simulink model "wlan_phy_rx_pmd"  (Simulink 7.8)
settings: Solver=VariableStepDiscrete  SolverMode=SingleTasking  StartTime=0.0  StopTime=rx_sim_time  RelTol=1e-3
PostLoadFcn:
  | wlan_phy_rx_init
InitFcn:
  | wlan_phy_rx_init
StartFcn:
  | wlan_phy_rx_init
values from neighbouring files (symbols this model uses):
  barker_seq20 = 0.95 * circshift(barker_seq20, [0 4]) ./ max(abs(barker_seq20))    [wlan_phy_rx_init.m]
  bit_scrambler_lfsr_bytes = sum(bit_scrambler_lfsr_bytes .* repmat(2.^[0:7], 127, 1),2)    [wlan_phy_rx_init.m]
  deinterleave_ROM = [deinterleave_ROM interleave_11n_64QAM zeros(1, 512-length(interleave_11n_64QAM))]    [wlan_phy_rx_init.m]
  longCorr_coef_i = [3*real(longCorr_coef)]    [wlan_phy_rx_init.m]
  longCorr_coef_q = [3*imag(longCorr_coef)]    [wlan_phy_rx_init.m]
  MAX_CP_LEN = 32    [wlan_phy_rx_init.m]
  MAX_NCBPS = NCBPS_11n_64QAM    (= 312)    [wlan_phy_rx_init.m]
  MAX_NUM_BYTES = 2^16-1    (= 65535)    [wlan_phy_rx_init.m]
  MAX_NUM_SC = 64    [wlan_phy_rx_init.m]
  MAX_NUM_SYMS = 600    [wlan_phy_rx_init.m]
  NCBPS_11a_BPSK = 48    [wlan_phy_rx_init.m]
  NDBPS_11a_BPSK12 = 24    [wlan_phy_rx_init.m]
  PHY_SIGNAL_MIN_LEN = 14    [wlan_phy_rx_init.m]
  preFFT_sampBuff_numSamps = 4*MAX_NUM_SC    (= 256)    [wlan_phy_rx_init.m]
  rx_sim_sig_samp_time = 8    [wlan_phy_rx_init.m]
  scr_ind_rev = zeros(1,128)    [wlan_phy_rx_init.m]
undefined symbols (not defined in the file or in its folder): gcbh, mcs_rom_11ag, mcs_rom_11n, xlBlockLoadCallback, xlGetNormalizedPeriod, xlipgetpartsetting
libraries: xbsIndex_r4 x3862, simulink x1, xbsEDKLib_r4 x1
(part 3 of 23)

== system wlan_phy_rx_pmd/FCS & Pkt Buf ==
  DSSS data : Inport
  OFDM data : Inport  Port=2
  Bus Selector : BusSelector  OutputSignals=byte_valid,byte_index,byte,last_byte
  Bus Selector1 : BusSelector  OutputSignals=byte_valid,byte_index,byte,last_byte
  Bytes In : Scope  NumInputPorts=8
      settings: TimeRange=10000  YMin=0~0~0~-1~-1~-1~-1~-1  YMax=1~90~225~1~1~1~1~1  SaveName=ScopeData36
  FCS Calc : Subsystem
  Gateway Out{1..4,8..9,11..12} (8 alike) : xbsIndex_r4/Gateway Out  inherit_from_input=off  hdl_port=off  timing_constraint=None  locs_specified=off  LOCs={}  UseAsDAC=off  DACChannel='1'  block_type=gatewayout
  Pkt Buf Interface : Subsystem
  wires:
  OFDM data/1 -> Bus Selector/1, Pkt Buf Interface/2(OFDM data), FCS Calc/2(OFDM data)
  DSSS data/1 -> Bus Selector1/1, Pkt Buf Interface/1(DSSS data), FCS Calc/1(DSSS data)
  Bus Selector1/1 -> Gateway Out9/1  "<byte_valid>"
  Bus Selector1/2 -> Gateway Out8/1  "<byte_index>"
  Bus Selector1/3 -> Gateway Out11/1  "<byte>"
  Bus Selector1/4 -> Gateway Out12/1  "<last_byte>"
  Bus Selector/1 -> Gateway Out1/1  "<byte_valid>"
  Bus Selector/2 -> Gateway Out2/1  "<byte_index>"
  Bus Selector/3 -> Gateway Out3/1  "<byte>"
  Bus Selector/4 -> Gateway Out4/1  "<last_byte>"
  Gateway Out9/1 -> Bytes In/1  "DSSS Valid"
  Gateway Out8/1 -> Bytes In/2  "DSSS Byte Index"
  Gateway Out11/1 -> Bytes In/3  "DSSS Byte"
  Gateway Out12/1 -> Bytes In/4  "DSSS Last Byte"
  Gateway Out1/1 -> Bytes In/5  "OFDM Valid"
  Gateway Out2/1 -> Bytes In/6  "OFDM Byte Index"
  Gateway Out3/1 -> Bytes In/7  "OFDM Byte"
  Gateway Out4/1 -> Bytes In/8  "OFDM Last Byte"

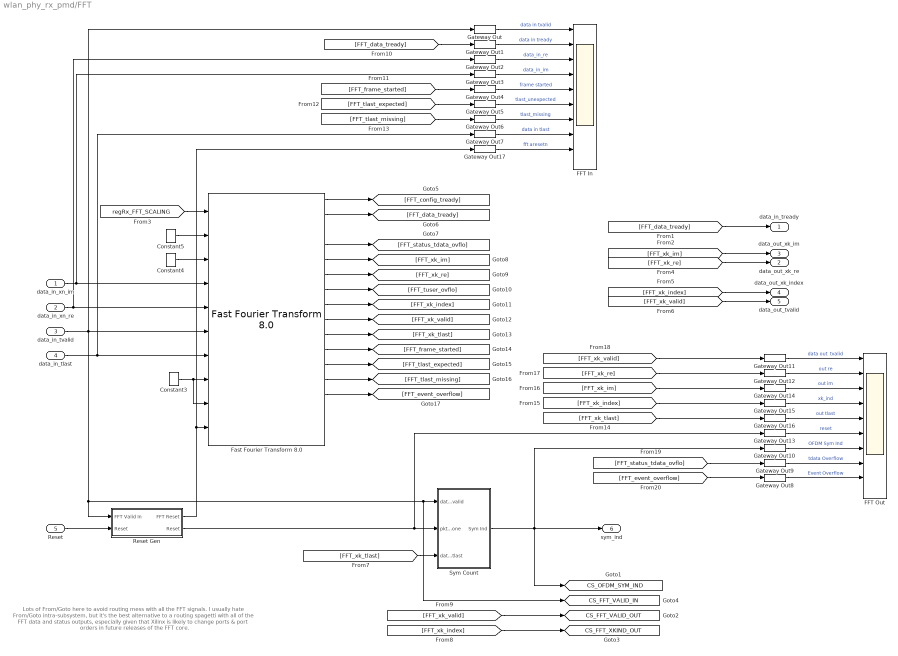
== system wlan_phy_rx_pmd/FFT ==
  note: Lots of From/Goto here to avoid routing
      | mess with all the FFT signals. I usually hate
      | From/Goto intra-subsystem, but it's the best
      | alternative to a routing spagetti with all of the
      | FFT data and status outputs, especially given
      | that Xilinx is likely to change ports & port orders
      | in future releases of the FFT core.
  data_in_xn_im : Inport
  data_in_xn_re : Inport  Port=2
  data_in_tvalid : Inport  Port=3
  data_in_tlast : Inport  Port=4
  Reset : Inport  Port=5
  Constant3 : xbsIndex_r4/Constant  const=1  gui_display_data_type=Boolean  arith_type=Boolean  n_bits=1  bin_pt=0  preci_type=Single  exp_width=8  frac_width=24  explicit_period=on  period=1  equ=P=C  opselect=C  inp2=PCIN>>17  opr=+  inp1=P  carry=CIN  dbl_ovrd=off  block_type=constant
  Constant4 : xbsIndex_r4/Constant  const=1  gui_display_data_type=Boolean  arith_type=Boolean  n_bits=1  bin_pt=0  preci_type=Single  exp_width=8  frac_width=24  explicit_period=on  period=1  equ=P=C  opselect=C  inp2=PCIN>>17  opr=+  inp1=P  carry=CIN  dbl_ovrd=off  block_type=constant
  Constant5 : xbsIndex_r4/Constant  const=1  gui_display_data_type=Fixed-point  arith_type=Unsigned  n_bits=1  bin_pt=0  preci_type=Single  exp_width=8  frac_width=24  explicit_period=on  period=1  equ=P=C  opselect=C  inp2=PCIN>>17  opr=+  inp1=P  carry=CIN  dbl_ovrd=off  block_type=constant
  FFT In : Scope  NumInputPorts=10
      settings: TimeRange=5000  YMin=0~0~-0.2~-1~0~-1~0~-1~-5~-5  YMax=1~1~0.8~1~1~1~1~1~5~5
  FFT Out : Scope  NumInputPorts=10
      settings: TimeRange=5000  YMin=0~-0.4~-0.4~0~0~-1~0~-1~-5~-5  YMax=1~0.4~0.4~70~1~1~1~1~5~5  SaveName=ScopeData29
  "Fast Fourier Transform 8.0 " : xbsIndex_r4/Fast Fourier Transform 8.0   transform_length=64  target_clock_frequency=160  target_data_throughput=50  implementation_options=radix_4_burst_io  run_time_configurable_transform_length=off  phase_factor_width=16  scaling_options=scaled  rounding_modes=truncation  aclken=off  aresetn=on  cyclic_prefix_insertion=off  output_ordering=natural_order  throttle_scheme=nonrealtime  xk_index=on  ovflo=on  trim_axipin_name=on  memory_options_data=block_ram  memory_options_phase_factors=block_ram  number_of_stages_using_block_ram_for_data_and_phase_factors=0  memory_options_reorder=block_ram  memory_options_hybrid=off  complex_mult_type=use_mults_performance  butterfly_type=use_luts  channels=1  input_ordering=natural_order  input_width=16  ip_name=Fast Fourier Transform  ip_version=8.0  dsptool_ready=true  ipcore_usecache=true  ipcore_useipmodelcache=true  wrapper_available=true  port_translation_map={ 'aclken' => 'en', 'aresetn' => 'rst'}  block_type=xfft_v8_0
  Gateway Out{,1..17} (18 alike) : xbsIndex_r4/Gateway Out  inherit_from_input=off  hdl_port=off  timing_constraint=None  locs_specified=off  LOCs={}  UseAsDAC=off  DACChannel='1'  block_type=gatewayout
  Reset Gen : Subsystem
  Sym Count : Subsystem
  data_in_tready : Outport
  data_out_xk_re : Outport  Port=2
  data_out_xk_im : Outport  Port=3
  data_out_xk_index : Outport  Port=4
  data_out_tvalid : Outport  Port=5
  sym_ind : Outport  Port=6
  tag scope: CS_FFT_VALID_IN=global, CS_FFT_VALID_OUT=global, CS_FFT_XKIND_OUT=global, CS_OFDM_SYM_IND=global
  wires:
  Gateway Out13/1 -> FFT Out/6  "reset"
  Gateway Out16/1 -> FFT Out/5  "out tlast"
  Gateway Out15/1 -> FFT Out/4  "xk_ind"
  Gateway Out14/1 -> FFT Out/3  "out im"
  Gateway Out12/1 -> FFT Out/2  "out re"
  Gateway Out11/1 -> FFT Out/1  "data out  tvalid"
  [FFT_xk_index] -> data_out_xk_index/1
  [FFT_xk_re] -> data_out_xk_re/1
  [FFT_xk_tlast] -> Sym Count/3(data_out_tlast)
  [FFT_xk_valid] -> data_out_tvalid/1
  [FFT_xk_im] -> data_out_xk_im/1
  [FFT_data_tready] -> data_in_tready/1
  Gateway Out7/1 -> FFT In/8  "data in tlast"
  Gateway Out6/1 -> FFT In/7  "tlast_missing"
  Gateway Out5/1 -> FFT In/6  "tlast_unexpected"
  Gateway Out4/1 -> FFT In/5  "frame started"
  Gateway Out3/1 -> FFT In/4  "data_in_im"
  Gateway Out2/1 -> FFT In/3  "data_in_re"
  Gateway Out1/1 -> FFT In/2  "data in tready"
  Gateway Out/1 -> FFT In/1  "data in tvalid"
  "Fast Fourier Transform 8.0 "/1 -> [FFT_config_tready]
  "Fast Fourier Transform 8.0 "/2 -> [FFT_data_tready]
  "Fast Fourier Transform 8.0 "/4 -> [FFT_status_tdata_ovflo]
  "Fast Fourier Transform 8.0 "/5 -> [FFT_xk_im]
  "Fast Fourier Transform 8.0 "/6 -> [FFT_xk_re]
  "Fast Fourier Transform 8.0 "/7 -> [FFT_tuser_ovflo]
  "Fast Fourier Transform 8.0 "/8 -> [FFT_xk_index]
  "Fast Fourier Transform 8.0 "/9 -> [FFT_xk_valid]
  "Fast Fourier Transform 8.0 "/10 -> [FFT_xk_tlast]
  "Fast Fourier Transform 8.0 "/11 -> [FFT_frame_started]
  "Fast Fourier Transform 8.0 "/12 -> [FFT_tlast_expected]
  "Fast Fourier Transform 8.0 "/13 -> [FFT_tlast_missing]
  "Fast Fourier Transform 8.0 "/14 -> [FFT_event_overflow]
  Constant3/1 -> "Fast Fourier Transform 8.0 "/8, "Fast Fourier Transform 8.0 "/9
  Constant4/1 -> "Fast Fourier Transform 8.0 "/3
  Constant5/1 -> "Fast Fourier Transform 8.0 "/2
  [regRx_FFT_SCALING] -> "Fast Fourier Transform 8.0 "/1
  [FFT_xk_index] -> [CS_FFT_XKIND_OUT]
  [FFT_xk_valid] -> [CS_FFT_VALID_OUT]
  Reset Gen/2(" Reset") -> Sym Count/2(pkt_done), Gateway Out13/1
  Reset/1 -> Reset Gen/2(Reset)
  data_in_tvalid/1 -> "Fast Fourier Transform 8.0 "/6, Reset Gen/1(FFT Valid In), Sym Count/1(data_in_tvalid), [CS_FFT_VALID_IN], Gateway Out/1
  Sym Count/1(Sym Ind) -> sym_ind/1, Gateway Out10/1, [CS_OFDM_SYM_IND]
  data_in_tlast/1 -> "Fast Fourier Transform 8.0 "/7, Gateway Out7/1
  data_in_xn_im/1 -> "Fast Fourier Transform 8.0 "/4, Gateway Out3/1
  data_in_xn_re/1 -> "Fast Fourier Transform 8.0 "/5, Gateway Out2/1
  Gateway Out10/1 -> FFT Out/7  "OFDM Sym Ind"
  Gateway Out9/1 -> FFT Out/8  "tdata Overflow"
  [FFT_data_tready] -> Gateway Out1/1
  [FFT_frame_started] -> Gateway Out4/1
  [FFT_tlast_expected] -> Gateway Out5/1
  [FFT_tlast_missing] -> Gateway Out6/1
  [FFT_xk_tlast] -> Gateway Out16/1
  [FFT_xk_index] -> Gateway Out15/1
  [FFT_xk_im] -> Gateway Out14/1
  [FFT_xk_re] -> Gateway Out12/1
  [FFT_xk_valid] -> Gateway Out11/1
  [FFT_status_tdata_ovflo] -> Gateway Out9/1
  [FFT_event_overflow] -> Gateway Out8/1
  Gateway Out8/1 -> FFT Out/9  "Event Overflow"
  Reset Gen/1(FFT Reset) -> "Fast Fourier Transform 8.0 "/10, Gateway Out17/1
  Gateway Out17/1 -> FFT In/9  "fft aresetn"

== system wlan_phy_rx_pmd/Inputs ==
  ADC_RX_CLK : xbsIndex_r4/Gateway In  gui_display_data_type=Boolean  arith_type=Boolean  n_bits=1  bin_pt=0  preci_type=Single  exp_width=8  frac_width=24  quantization="Round  (unbiased: +/- Inf)"  overflow=Saturate  period=1  dbl_ovrd=off  timing_constraint=None  locs_specified=off  LOCs={}  UseAsADC=off  ADCChannel='1'  inherit_from_input=off  hdl_port=on  block_type=gatewayin
  AGC_DONE : xbsIndex_r4/Gateway In  gui_display_data_type=Boolean  arith_type=Boolean  n_bits=5  bin_pt=0  preci_type=Single  exp_width=8  frac_width=24  quantization="Round  (unbiased: +/- Inf)"  overflow=Saturate  period=1  dbl_ovrd=off  timing_constraint=None  locs_specified=off  LOCs={}  UseAsADC=off  ADCChannel='1'  inherit_from_input=off  hdl_port=on  block_type=gatewayin
  Bus Creator{,1..3} (4 alike) : BusCreator
  Constant : Constant  Value=0
  Constant1 : Constant
  Convert2 : xbsIndex_r4/Convert  gui_display_data_type=Boolean  arith_type=Boolean  n_bits=1  bin_pt=0  float_type=Single  exp_bits=8  fraction_bits=24  quantization=Truncate  overflow=Wrap  en=off  latency=0  dbl_ovrd=off  pipeline=off  block_type=convert
  Delay3 : xbsIndex_r4/Delay  rst=off  infoeditControl=Selection of Reset will increase slice count due to use of real FFs and instead of SRLs  en=off  latency=1  dbl_ovrd=off  reg_retiming=off  block_type=delay
  Logical : xbsIndex_r4/Logical  logical_function=AND  inputs=2  en=off  latency=0  precision=Full  arith_type=Unsigned  n_bits=16  bin_pt=0  align_bp=on  dbl_ovrd=off  block_type=logical
  Pulse Generator3 : DiscretePulseGenerator  Period=8
  RF A Inputs : Subsystem
  RF B Inputs : Subsystem
  RF C Inputs : Subsystem
  RF D Inputs : Subsystem
  RSSI Clock Gen : Subsystem
  RSSI_ADC_CLK : xbsIndex_r4/Gateway Out  inherit_from_input=off  hdl_port=on  timing_constraint=None  locs_specified=off  LOCs={}  UseAsDAC=off  DACChannel='1'  block_type=gatewayout
      settings: infoedit="Gateway out block.  Converts Xilinx fixed point inputs into ouputs of type Simulink integer, double, or fixed point.<P><P>Hardware notes:  In hardware these blocks become top level output ports or are discarded, depending on how they are configured."  block_version=8.2
  RX_SIGS_INVALID : xbsIndex_r4/Gateway In  gui_display_data_type=Boolean  arith_type=Boolean  n_bits=1  bin_pt=0  preci_type=Single  exp_width=8  frac_width=24  quantization="Round  (unbiased: +/- Inf)"  overflow=Saturate  period=1  dbl_ovrd=off  timing_constraint=None  locs_specified=off  LOCs={}  UseAsADC=off  ADCChannel='1'  inherit_from_input=off  hdl_port=on  block_type=gatewayin
  Register3 : xbsIndex_r4/Register  init=0  rst=off  en=off  dbl_ovrd=off  block_type=register
  Register6 : xbsIndex_r4/Register  init=0  rst=off  en=off  dbl_ovrd=off  block_type=register
  Terminator : Terminator
  negedge1 : Subsystem
  ADC I : Outport
  ADC Q : Outport  Port=2
  IQ Valid : Outport  Port=3
  RSSI : Outport  Port=4
  tag scope: AGC_DONE=global, CS_ADC_IQ_Valid=global, CS_RX_SIGS_INVALID=global
  wires:
  RSSI_ADC_CLK/1 -> Terminator/1
  RSSI Clock Gen/1(Clk) -> RSSI_ADC_CLK/1
  Constant/1 -> RX_SIGS_INVALID/1
  RX_SIGS_INVALID/1 -> Register6/1
  Logical/1 -> [CS_RX_SIGS_INVALID], RF A Inputs/1(Rst), RF B Inputs/1(Rst), RF C Inputs/1(Rst), RF D Inputs/1(Rst)
  Register6/1 -> Logical/2
  [REG_USE_RX_SIGS_INVALID_INPUT] -> Logical/1
  Bus Creator/1 -> ADC I/1
  Bus Creator1/1 -> ADC Q/1
  Bus Creator2/1 -> RSSI/1
  [RFA_ADC_I] -> Bus Creator/1  "RFA_I"
  [RFB_ADC_I] -> Bus Creator/2  "RFB_I"
  [RFA_ADC_Q] -> Bus Creator1/1  "RFA_Q"
  [RFB_ADC_Q] -> Bus Creator1/2  "RFB_Q"
  [RFA_RSSI] -> Bus Creator2/1  "RFA_RSSI"
  [RFB_RSSI] -> Bus Creator2/2  "RFB_RSSI"
  AGC_DONE/1 -> Delay3/1
  Delay3/1 -> [AGC_DONE]
  Bus Creator3/1 -> IQ Valid/1
  Convert2/1 -> [CS_ADC_IQ_Valid], RF A Inputs/2(IQ Valid), Bus Creator3/1, Bus Creator3/2, Bus Creator3/3, Bus Creator3/4, RF B Inputs/2(IQ Valid), RF C Inputs/2(IQ Valid), RF D Inputs/2(IQ Valid)
  negedge1/1(Q) -> Convert2/1
  Register3/1 -> negedge1/1(D)
  ADC_RX_CLK/1 -> Register3/1
  Pulse Generator3/1 -> ADC_RX_CLK/1
  RF A Inputs/1(ADC I) -> [RFA_ADC_I]
  RF A Inputs/2(ADC Q) -> [RFA_ADC_Q]
  RF A Inputs/3(Avg RSSI) -> [RFA_RSSI]
  RF B Inputs/1(ADC I) -> [RFB_ADC_I]
  RF B Inputs/2(ADC Q) -> [RFB_ADC_Q]
  RF B Inputs/3(Avg RSSI) -> [RFB_RSSI]
  [RFD_ADC_I] -> Bus Creator/4  "RFD_I"
  [RFC_ADC_I] -> Bus Creator/3  "RFC_I"
  [RFC_ADC_Q] -> Bus Creator1/3  "RFC_Q"
  [RFD_ADC_Q] -> Bus Creator1/4  "RFD_Q"
  [RFC_RSSI] -> Bus Creator2/3  "RFC_RSSI"
  [RFD_RSSI] -> Bus Creator2/4  "RFD_RSSI"
  RF C Inputs/1(ADC I) -> [RFC_ADC_I]
  RF C Inputs/2(ADC Q) -> [RFC_ADC_Q]
  RF C Inputs/3(Avg RSSI) -> [RFC_RSSI]
  RF D Inputs/1(ADC I) -> [RFD_ADC_I]
  RF D Inputs/2(ADC Q) -> [RFD_ADC_Q]
  RF D Inputs/3(Avg RSSI) -> [RFD_RSSI]
  Constant1/1 -> AGC_DONE/1

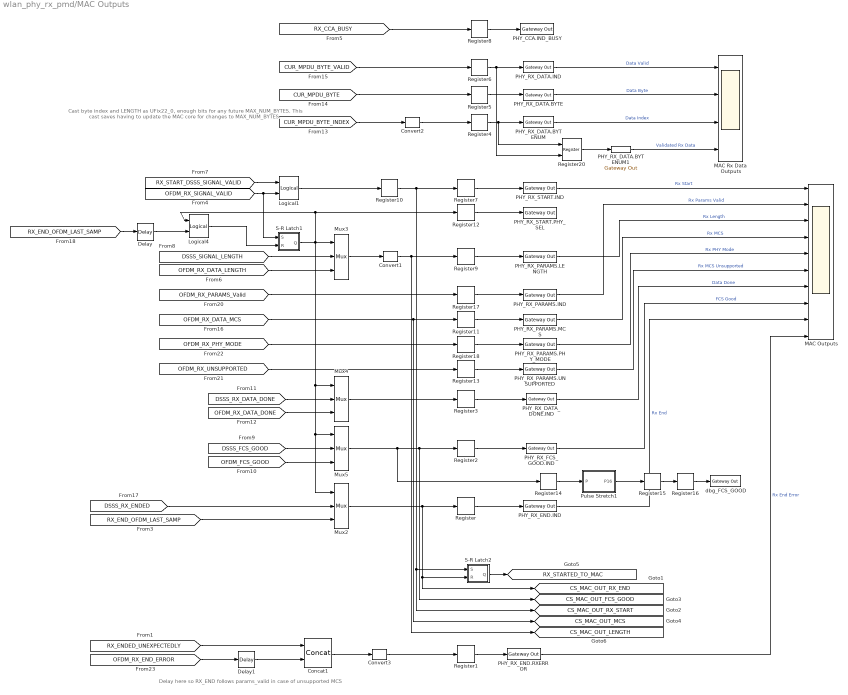
== system wlan_phy_rx_pmd/MAC Outputs ==
  note: Cast byte index and LENGTH as UFix22_0, enough bits
      | for any future MAX_NUM_BYTES. This cast saves having to
      | update the MAC core for changes to MAX_NUM_BYTES.
  note: Delay here so RX_END follows params_valid
      | in case of unsupported MCS
  Concat1 : xbsIndex_r4/Concat  num_inputs=2  dbl_ovrd=off  block_type=concat
  Convert1 : xbsIndex_r4/Convert  gui_display_data_type=Fixed-point  arith_type=Unsigned  n_bits=ceil(log2(MAX_NUM_BYTES))  bin_pt=0  float_type=Single  exp_bits=8  fraction_bits=24  quantization=Truncate  overflow=Wrap  en=off  latency=0  dbl_ovrd=off  pipeline=off  block_type=convert
  Convert2 : xbsIndex_r4/Convert  gui_display_data_type=Fixed-point  arith_type=Unsigned  n_bits=ceil(log2(MAX_NUM_BYTES))  bin_pt=0  float_type=Single  exp_bits=8  fraction_bits=24  quantization=Truncate  overflow=Wrap  en=off  latency=0  dbl_ovrd=off  pipeline=off  block_type=convert
  Convert3 : xbsIndex_r4/Convert  gui_display_data_type=Fixed-point  arith_type=Unsigned  n_bits=4  bin_pt=0  float_type=Single  exp_bits=8  fraction_bits=24  quantization=Truncate  overflow=Wrap  en=off  latency=0  dbl_ovrd=off  pipeline=off  block_type=convert
  Delay : xbsIndex_r4/Delay  rst=off  infoeditControl=Selection of Reset will increase slice count due to use of real FFs and instead of SRLs  en=off  latency=8  dbl_ovrd=off  reg_retiming=off  block_type=delay
  Delay1 : xbsIndex_r4/Delay  rst=off  infoeditControl=Selection of Reset will increase slice count due to use of real FFs and instead of SRLs  en=off  latency=2  dbl_ovrd=off  reg_retiming=off  block_type=delay
  Logical1 : xbsIndex_r4/Logical  logical_function=OR  inputs=2  en=off  latency=0  precision=Full  arith_type=Unsigned  n_bits=16  bin_pt=0  align_bp=on  dbl_ovrd=off  block_type=logical
  Logical4 : xbsIndex_r4/Logical  logical_function=AND  inputs=2  en=off  latency=0  precision=Full  arith_type=Unsigned  n_bits=16  bin_pt=0  align_bp=on  dbl_ovrd=off  block_type=logical
  MAC Outputs : Scope  NumInputPorts=10
      settings: TimeRange=9380  YMin=0~0~0~0~0~-1~0~0~0~-1  YMax=1~1~150~4~1~1~1~1~1~1  SaveName=ScopeData37
  MAC Rx Data Outputs : Scope  NumInputPorts=4
      settings: TimeRange=70000  YMin=0~0~0~0  YMax=1~1~150~8  SaveName=ScopeData32
  Mux{2..5} (4 alike) : xbsIndex_r4/Mux  inputs=2  en=off  latency=0  precision=Full  arith_type=Unsigned  n_bits=16  bin_pt=14  quantization=Truncate  overflow=Wrap  dbl_ovrd=off  block_type=mux
  PHY_CCA.IND_BUSY : xbsIndex_r4/Gateway Out  inherit_from_input=off  hdl_port=on  timing_constraint=None  locs_specified=off  LOCs={}  UseAsDAC=off  DACChannel='1'  block_type=gatewayout
  PHY_RX_DATA.BYTE : xbsIndex_r4/Gateway Out  inherit_from_input=off  hdl_port=on  timing_constraint=None  locs_specified=off  LOCs={}  UseAsDAC=off  DACChannel='1'  block_type=gatewayout
  PHY_RX_DATA.BYTENUM : xbsIndex_r4/Gateway Out  inherit_from_input=off  hdl_port=on  timing_constraint=None  locs_specified=off  LOCs={}  UseAsDAC=off  DACChannel='1'  block_type=gatewayout
  PHY_RX_DATA.BYTENUM1 : xbsIndex_r4/Gateway Out  inherit_from_input=off  hdl_port=off  timing_constraint=None  locs_specified=off  LOCs={}  UseAsDAC=off  DACChannel='1'  block_type=gatewayout
  PHY_RX_DATA.IND : xbsIndex_r4/Gateway Out  inherit_from_input=off  hdl_port=on  timing_constraint=None  locs_specified=off  LOCs={}  UseAsDAC=off  DACChannel='1'  block_type=gatewayout
  PHY_RX_DATA_DONE.IND : xbsIndex_r4/Gateway Out  inherit_from_input=off  hdl_port=on  timing_constraint=None  locs_specified=off  LOCs={}  UseAsDAC=off  DACChannel='1'  block_type=gatewayout
  PHY_RX_END.IND : xbsIndex_r4/Gateway Out  inherit_from_input=off  hdl_port=on  timing_constraint=None  locs_specified=off  LOCs={}  UseAsDAC=off  DACChannel='1'  block_type=gatewayout
  PHY_RX_END.RXERROR : xbsIndex_r4/Gateway Out  inherit_from_input=off  hdl_port=on  timing_constraint=None  locs_specified=off  LOCs={}  UseAsDAC=off  DACChannel='1'  block_type=gatewayout
  PHY_RX_FCS_GOOD.IND : xbsIndex_r4/Gateway Out  inherit_from_input=off  hdl_port=on  timing_constraint=None  locs_specified=off  LOCs={}  UseAsDAC=off  DACChannel='1'  block_type=gatewayout
  PHY_RX_PARAMS.IND : xbsIndex_r4/Gateway Out  inherit_from_input=off  hdl_port=on  timing_constraint=None  locs_specified=off  LOCs={}  UseAsDAC=off  DACChannel='1'  block_type=gatewayout
  PHY_RX_PARAMS.LENGTH : xbsIndex_r4/Gateway Out  inherit_from_input=off  hdl_port=on  timing_constraint=None  locs_specified=off  LOCs={}  UseAsDAC=off  DACChannel='1'  block_type=gatewayout
  PHY_RX_PARAMS.MCS : xbsIndex_r4/Gateway Out  inherit_from_input=off  hdl_port=on  timing_constraint=None  locs_specified=off  LOCs={}  UseAsDAC=off  DACChannel='1'  block_type=gatewayout
  PHY_RX_PARAMS.PHY_MODE : xbsIndex_r4/Gateway Out  inherit_from_input=off  hdl_port=on  timing_constraint=None  locs_specified=off  LOCs={}  UseAsDAC=off  DACChannel='1'  block_type=gatewayout
  PHY_RX_PARAMS.UNSUPPORTED : xbsIndex_r4/Gateway Out  inherit_from_input=off  hdl_port=on  timing_constraint=None  locs_specified=off  LOCs={}  UseAsDAC=off  DACChannel='1'  block_type=gatewayout
  PHY_RX_START.IND : xbsIndex_r4/Gateway Out  inherit_from_input=off  hdl_port=on  timing_constraint=None  locs_specified=off  LOCs={}  UseAsDAC=off  DACChannel='1'  block_type=gatewayout
  PHY_RX_START.PHY_SEL : xbsIndex_r4/Gateway Out  inherit_from_input=off  hdl_port=on  timing_constraint=None  locs_specified=off  LOCs={}  UseAsDAC=off  DACChannel='1'  block_type=gatewayout
  Pulse Stretch1 : Subsystem
  Register{,1..18} (19 alike) : xbsIndex_r4/Register  init=0  rst=off  en=off  dbl_ovrd=off  block_type=register
  Register20 : xbsIndex_r4/Register  init=0  rst=off  en=on  dbl_ovrd=off  block_type=register
  S-R Latch1 : Subsystem
  S-R Latch2 : Subsystem
  dbg_FCS_GOOD : xbsIndex_r4/Gateway Out  inherit_from_input=off  hdl_port=on  timing_constraint=None  locs_specified=off  LOCs={}  UseAsDAC=off  DACChannel='1'  block_type=gatewayout
  tag scope: CS_MAC_OUT_FCS_GOOD=global, CS_MAC_OUT_LENGTH=global, CS_MAC_OUT_MCS=global, CS_MAC_OUT_RX_END=global, CS_MAC_OUT_RX_START=global, RX_STARTED_TO_MAC=global
  wires:
  Register/1 -> PHY_RX_END.IND/1
  Register1/1 -> PHY_RX_END.RXERROR/1
  Register2/1 -> PHY_RX_FCS_GOOD.IND/1
  Register3/1 -> PHY_RX_DATA_DONE.IND/1
  [CUR_MPDU_BYTE] -> Register5/1
  [CUR_MPDU_BYTE_VALID] -> Register6/1
  [CUR_MPDU_BYTE_INDEX] -> Convert2/1
  Register4/1 -> PHY_RX_DATA.BYTENUM/1, Register20/1
  Register5/1 -> PHY_RX_DATA.BYTE/1
  Register6/1 -> PHY_RX_DATA.IND/1, Register20/2
  [RX_CCA_BUSY] -> Register8/1
  Register7/1 -> PHY_RX_START.IND/1
  Register8/1 -> PHY_CCA.IND_BUSY/1
  Register9/1 -> PHY_RX_PARAMS.LENGTH/1
  [DSSS_FCS_GOOD] -> Mux5/2
  [OFDM_FCS_GOOD] -> Mux5/3
  [DSSS_RX_DATA_DONE] -> Mux4/2
  [OFDM_RX_DATA_DONE] -> Mux4/3
  Mux4/1 -> Register3/1
  [RX_START_DSSS_SIGNAL_VALID] -> Logical1/1
  [OFDM_RX_SIGNAL_VALID] -> Logical1/2, S-R Latch1/1(S)
  Logical1/1 -> Register10/1
  S-R Latch1/1(Q) -> Mux3/1, Logical4/1, Register12/1, Mux2/1, Mux5/1, Mux4/1
  [OFDM_RX_DATA_LENGTH] -> Mux3/3
  [DSSS_SIGNAL_LENGTH] -> Mux3/2
  Mux3/1 -> Convert1/1
  Logical4/1 -> S-R Latch1/2(R)
  [RX_END_OFDM_LAST_SAMP] -> Mux2/3
  Concat1/1 -> Convert3/1
  Mux2/1 -> Register/1, [CS_MAC_OUT_RX_END], S-R Latch2/2(R)
  [DSSS_RX_ENDED] -> Mux2/2
  Mux5/1 -> Register14/1, Register2/1, [CS_MAC_OUT_FCS_GOOD]
  [RX_END_OFDM_LAST_SAMP] -> Delay/1
  Register10/1 -> Register7/1, [CS_MAC_OUT_RX_START], S-R Latch2/1(S)
  Delay/1 -> Logical4/2
  Register14/1 -> Pulse Stretch1/1(P)
  Register15/1 -> Register16/1
  Register16/1 -> dbg_FCS_GOOD/1
  S-R Latch2/1(Q) -> [RX_STARTED_TO_MAC]
  Pulse Stretch1/1(P16) -> Register15/1
  Convert2/1 -> Register4/1
  Register12/1 -> PHY_RX_START.PHY_SEL/1
  Register11/1 -> PHY_RX_PARAMS.MCS/1
  [OFDM_RX_DATA_MCS] -> Register11/1, [CS_MAC_OUT_MCS]
  Register13/1 -> PHY_RX_PARAMS.UNSUPPORTED/1
  [OFDM_RX_UNSUPPORTED] -> Register13/1
  [OFDM_RX_PARAMS_Valid] -> Register17/1
  Register17/1 -> PHY_RX_PARAMS.IND/1
  Register18/1 -> PHY_RX_PARAMS.PHY_MODE/1
  [OFDM_RX_PHY_MODE] -> Register18/1
  PHY_RX_START.IND/1 -> MAC Outputs/1  "Rx Start"
  PHY_RX_PARAMS.IND/1 -> MAC Outputs/2  "Rx Params Valid"
  PHY_RX_PARAMS.LENGTH/1 -> MAC Outputs/3  "Rx Length"
  PHY_RX_PARAMS.MCS/1 -> MAC Outputs/4  "Rx MCS"
  PHY_RX_PARAMS.PHY_MODE/1 -> MAC Outputs/5  "Rx PHY Mode"
  PHY_RX_PARAMS.UNSUPPORTED/1 -> MAC Outputs/6  "Rx MCS Unsupported"
  PHY_RX_DATA_DONE.IND/1 -> MAC Outputs/7  "Data Done"
  PHY_RX_FCS_GOOD.IND/1 -> MAC Outputs/8  "FCS Good"
  PHY_RX_END.IND/1 -> MAC Outputs/9  "Rx End"
  PHY_RX_END.RXERROR/1 -> MAC Outputs/10  "Rx End Error"
  Convert1/1 -> Register9/1, [CS_MAC_OUT_LENGTH]
  Convert3/1 -> Register1/1
  PHY_RX_DATA.IND/1 -> MAC Rx Data Outputs/1  "Data Valid"
  PHY_RX_DATA.BYTE/1 -> MAC Rx Data Outputs/2  "Data Byte"
  PHY_RX_DATA.BYTENUM/1 -> MAC Rx Data Outputs/3  "Data Index"
  Register20/1 -> PHY_RX_DATA.BYTENUM1/1
  PHY_RX_DATA.BYTENUM1/1 -> MAC Rx Data Outputs/4  "Validated Rx Data"
  [RX_ENDED_UNEXPECTEDLY] -> Concat1/1
  [OFDM_RX_END_ERROR] -> Delay1/1
  Delay1/1 -> Concat1/2

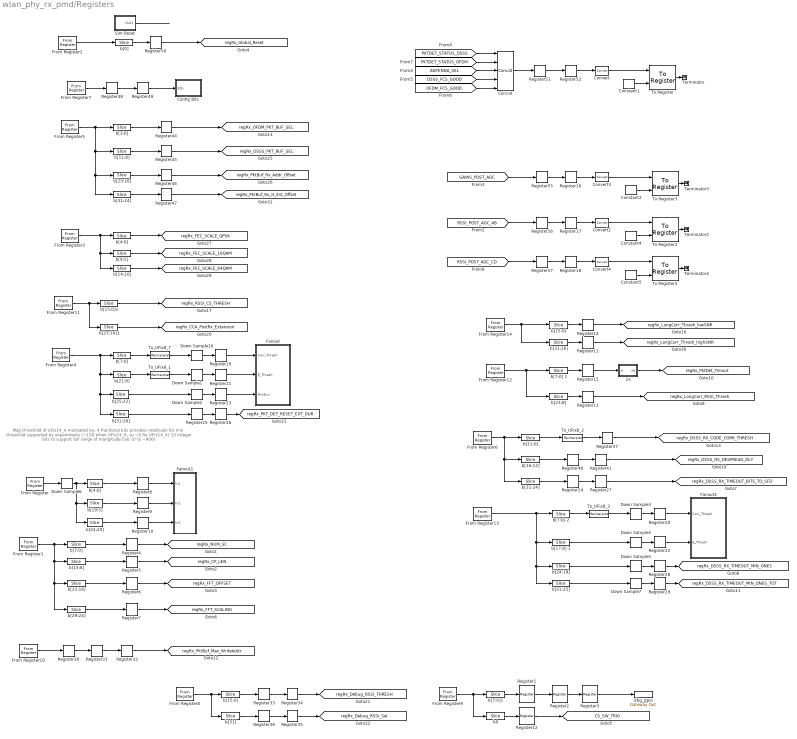
== system wlan_phy_rx_pmd/Registers ==
  note: Mag threshold of UFix14_4 motivated by:
      | 4 fractional bits provides resoltuion for min threshold supported by experiments (~150 when UFix14_8, so ~9 for UFix14_4)
      | 10 integer bits to support full range of mangitude calc (0 to ~900)
  " b[23:16] " : xbsIndex_r4/Slice  nbits=8  boolean_output=off  mode=Lower Bit Location + Width  bit1=0  base1=MSB of Input  bit0=16  base0=LSB of Input  dbl_ovrd=off  block_type=slice
      settings: block_version=VER_STRING_GOES_HERE
  " b[31:24]" : xbsIndex_r4/Slice  nbits=8  boolean_output=off  mode=Lower Bit Location + Width  bit1=0  base1=MSB of Input  bit0=24  base0=LSB of Input  dbl_ovrd=off  block_type=slice
      settings: block_version=VER_STRING_GOES_HERE
  "2x " : Subsystem
  Concat : xbsIndex_r4/Concat  num_inputs=5  dbl_ovrd=off  block_type=concat
      settings: block_version=8.2.02
  Config Bits : Subsystem
  Constant{1..2,4..5} (4 alike) : xbsIndex_r4/Constant  const=1  gui_display_data_type=Boolean  arith_type=Boolean  n_bits=30  bin_pt=0  preci_type=Single  exp_width=8  frac_width=24  explicit_period=on  period=1  equ=P=C  opselect=C  inp2=PCIN>>17  opr=+  inp1=P  carry=CIN  dbl_ovrd=off  block_type=constant
  Convert{,2..4} (4 alike) : xbsIndex_r4/Convert  gui_display_data_type=Fixed-point  arith_type=Unsigned  n_bits=32  bin_pt=0  float_type=Single  exp_bits=8  fraction_bits=24  quantization=Truncate  overflow=Wrap  en=off  latency=0  dbl_ovrd=off  pipeline=off  block_type=convert
  Down Sample{1..5,7,16} (7 alike) : xbsIndex_r4/Down Sample  sample_ratio=8  sample_phase="Last Value of Frame  (most efficient)"  en=off  latency=1  dbl_ovrd=off  block_type=dsamp
      settings: block_version=VER_STRING_GOES_HERE
  Down Sample6 : xbsIndex_r4/Down Sample  sample_ratio=16  sample_phase="Last Value of Frame  (most efficient)"  en=off  latency=1  dbl_ovrd=off  block_type=dsamp
      settings: block_version=VER_STRING_GOES_HERE
  Fanout : Subsystem
  Fanout1 : Subsystem
  Fanout2 : Subsystem
  From Register : xbsIndex_r4/From Register  shared_memory_name='PKTDET_RSSI_CONFIG'  init=REG_RX_PKTDET_RSSI_CONFIG  period=1  ownership=Locally owned and initialized  gui_display_data_type=Fixed-point  arith_type=Unsigned  n_bits=32  bin_pt=0  preci_type=Single  dbl_ovrd=off  block_type=fromreg
      settings: AttributesFormatString=<< %<shared_memory_name> >>  infoedit="Register block that reads data to a shared memory register.  Delay of one sample period."
  From Register1 : xbsIndex_r4/From Register  shared_memory_name='FFT_Config'  init=REG_RX_FFT_Config  period=1  ownership=Locally owned and initialized  gui_display_data_type=Fixed-point  arith_type=Unsigned  n_bits=32  bin_pt=0  preci_type=Single  dbl_ovrd=off  block_type=fromreg
      settings: AttributesFormatString=<< %<shared_memory_name> >>  infoedit="Register block that reads data to a shared memory register.  Delay of one sample period."
  From Register10 : xbsIndex_r4/From Register  shared_memory_name='PKTBUF_MAX_WRITE_ADDR'  init=REG_RX_PKT_BUF_Max_Write_Addr  period=1  ownership=Locally owned and initialized  gui_display_data_type=Fixed-point  arith_type=Unsigned  n_bits=32  bin_pt=0  preci_type=Single  dbl_ovrd=off  block_type=fromreg
      settings: AttributesFormatString=<< %<shared_memory_name> >>  infoedit="Register block that reads data to a shared memory register.  Delay of one sample period."
  From Register11 : xbsIndex_r4/From Register  shared_memory_name='PHY_CCA_CONFIG'  init=REG_RX_CCA_CONFIG  period=1  ownership=Locally owned and initialized  gui_display_data_type=Fixed-point  arith_type=Unsigned  n_bits=32  bin_pt=0  preci_type=Single  dbl_ovrd=off  block_type=fromreg
      settings: AttributesFormatString=<< %<shared_memory_name> >>  infoedit="Register block that reads data to a shared memory register.  Delay of one sample period."
  From Register12 : xbsIndex_r4/From Register  shared_memory_name='LTS_Corr_Config'  init=REG_RX_LTS_Corr_Confg  period=1  ownership=Locally owned and initialized  gui_display_data_type=Fixed-point  arith_type=Unsigned  n_bits=32  bin_pt=0  preci_type=Single  dbl_ovrd=off  block_type=fromreg
      settings: AttributesFormatString=<< %<shared_memory_name> >>  infoedit="Register block that reads data to a shared memory register.  Delay of one sample period."
  From Register13 : xbsIndex_r4/From Register  shared_memory_name='PKTDET_DSSS_CONFIG'  init=REG_RX_PktDet_DSSS_Config  period=1  ownership=Locally owned and initialized  gui_display_data_type=Fixed-point  arith_type=Unsigned  n_bits=32  bin_pt=0  preci_type=Single  dbl_ovrd=off  block_type=fromreg
      settings: AttributesFormatString=<< %<shared_memory_name> >>  infoedit="Register block that reads data to a shared memory register.  Delay of one sample period."
  From Register14 : xbsIndex_r4/From Register  shared_memory_name='LTS_Corr_Thresh'  init=REG_RX_LTS_Corr_Thresh  period=1  ownership=Locally owned and initialized  gui_display_data_type=Fixed-point  arith_type=Unsigned  n_bits=32  bin_pt=0  preci_type=Single  dbl_ovrd=off  block_type=fromreg
      settings: AttributesFormatString=<< %<shared_memory_name> >>  infoedit="Register block that reads data to a shared memory register.  Delay of one sample period."
  From Register2 : xbsIndex_r4/From Register  shared_memory_name='Control'  init=REG_RX_Control  period=1  ownership=Locally owned and initialized  gui_display_data_type=Fixed-point  arith_type=Unsigned  n_bits=32  bin_pt=0  preci_type=Single  dbl_ovrd=off  block_type=fromreg
      settings: AttributesFormatString=<< %<shared_memory_name> >>  infoedit="Register block that reads data to a shared memory register.  Delay of one sample period."
  From Register3 : xbsIndex_r4/From Register  shared_memory_name='FEC_CONFIG'  init=REG_RX_FEC_Config  period=1  ownership=Locally owned and initialized  gui_display_data_type=Fixed-point  arith_type=Unsigned  n_bits=32  bin_pt=0  preci_type=Single  dbl_ovrd=off  block_type=fromreg
      settings: AttributesFormatString=<< %<shared_memory_name> >>  infoedit="Register block that reads data to a shared memory register.  Delay of one sample period."
  From Register4 : xbsIndex_r4/From Register  shared_memory_name='PKTDET_AUTOCORR_CONFIG'  init=REG_RX_PktDet_AutoCorr_Config  period=1  ownership=Locally owned and initialized  gui_display_data_type=Fixed-point  arith_type=Unsigned  n_bits=32  bin_pt=0  preci_type=Single  dbl_ovrd=off  block_type=fromreg
      settings: AttributesFormatString=<< %<shared_memory_name> >>  infoedit="Register block that reads data to a shared memory register.  Delay of one sample period."
  From Register5 : xbsIndex_r4/From Register  shared_memory_name='PKT_BUF_SEL'  init=REG_RX_PktBuf_Sel  period=1  ownership=Locally owned and initialized  gui_display_data_type=Fixed-point  arith_type=Unsigned  n_bits=32  bin_pt=0  preci_type=Single  dbl_ovrd=off  block_type=fromreg
      settings: AttributesFormatString=<< %<shared_memory_name> >>  infoedit="Register block that reads data to a shared memory register.  Delay of one sample period."
  From Register6 : xbsIndex_r4/From Register  shared_memory_name='DSSS_RX_CONFIG'  init=REG_RX_DSSS_RX_CONFIG  period=1  ownership=Locally owned and initialized  gui_display_data_type=Fixed-point  arith_type=Unsigned  n_bits=32  bin_pt=0  preci_type=Single  dbl_ovrd=off  block_type=fromreg
      settings: AttributesFormatString=<< %<shared_memory_name> >>  infoedit="Register block that reads data to a shared memory register.  Delay of one sample period."
  From Register7 : xbsIndex_r4/From Register  shared_memory_name='CONFIG'  init=REG_RX_Config  period=1  ownership=Locally owned and initialized  gui_display_data_type=Fixed-point  arith_type=Unsigned  n_bits=32  bin_pt=0  preci_type=Single  dbl_ovrd=off  block_type=fromreg
      settings: AttributesFormatString=<< %<shared_memory_name> >>  infoedit="Register block that reads data to a shared memory register.  Delay of one sample period."
  From Register8 : xbsIndex_r4/From Register  shared_memory_name='RSSI_THRESH'  init=600  period=1  ownership=Locally owned and initialized  gui_display_data_type=Fixed-point  arith_type=Unsigned  n_bits=32  bin_pt=0  preci_type=Single  dbl_ovrd=off  block_type=fromreg
      settings: AttributesFormatString=<< %<shared_memory_name> >>  infoedit="Register block that reads data to a shared memory register.  Delay of one sample period."
  From Register9 : xbsIndex_r4/From Register  shared_memory_name='DEBUG_GPIO'  init=0  period=1  ownership=Locally owned and initialized  gui_display_data_type=Fixed-point  arith_type=Unsigned  n_bits=32  bin_pt=0  preci_type=Single  dbl_ovrd=off  block_type=fromreg
      settings: AttributesFormatString=<< %<shared_memory_name> >>  infoedit="Register block that reads data to a shared memory register.  Delay of one sample period."
  Register{1..37,40..41,44..52,55..57} (51 alike) : xbsIndex_r4/Register  init=0  rst=off  en=off  dbl_ovrd=off  block_type=register
  Sim Reset : Subsystem
  Terminator{,2..4} (4 alike) : Terminator
  To Register : xbsIndex_r4/To Register  shared_memory_name='Status'  init=0  ownership=Locally owned and initialized  explicit_data_type=on  gui_display_data_type=Fixed-point  arith_type=Unsigned  n_bits=32  bin_pt=0  preci_type=Single  dbl_ovrd=off  block_type=toreg
      settings: AttributesFormatString=<< %<shared_memory_name> >>  infoedit="Register block that writes data to a shared memory register.  Delay of one sample period."
  To Register2 : xbsIndex_r4/To Register  shared_memory_name='RX_PKT_RSSI_AB'  init=0  ownership=Locally owned and initialized  explicit_data_type=on  gui_display_data_type=Fixed-point  arith_type=Unsigned  n_bits=32  bin_pt=0  preci_type=Single  dbl_ovrd=off  block_type=toreg
      settings: AttributesFormatString=<< %<shared_memory_name> >>  infoedit="Register block that writes data to a shared memory register.  Delay of one sample period."
  To Register3 : xbsIndex_r4/To Register  shared_memory_name='RX_PKT_AGC_GAINS'  init=0  ownership=Locally owned and initialized  explicit_data_type=on  gui_display_data_type=Fixed-point  arith_type=Unsigned  n_bits=32  bin_pt=0  preci_type=Single  dbl_ovrd=off  block_type=toreg
      settings: AttributesFormatString=<< %<shared_memory_name> >>  infoedit="Register block that writes data to a shared memory register.  Delay of one sample period."
  To Register4 : xbsIndex_r4/To Register  shared_memory_name='RX_PKT_RSSI_CD'  init=0  ownership=Locally owned and initialized  explicit_data_type=on  gui_display_data_type=Fixed-point  arith_type=Unsigned  n_bits=32  bin_pt=0  preci_type=Single  dbl_ovrd=off  block_type=toreg
      settings: AttributesFormatString=<< %<shared_memory_name> >>  infoedit="Register block that writes data to a shared memory register.  Delay of one sample period."
  To_UFix8_1 : xbsIndex_r4/Reinterpret  force_arith_type=on  arith_type=Unsigned  force_bin_pt=on  bin_pt=4  block_type=cast
      settings: block_version=VER_STRING_GOES_HERE
  To_UFix8_2 : xbsIndex_r4/Reinterpret  force_arith_type=on  arith_type=Unsigned  force_bin_pt=on  bin_pt=4  block_type=cast
      settings: block_version=VER_STRING_GOES_HERE
  To_UFix8_3 : xbsIndex_r4/Reinterpret  force_arith_type=on  arith_type=Unsigned  force_bin_pt=on  bin_pt=6  block_type=cast
      settings: block_version=VER_STRING_GOES_HERE
  To_UFix8_7 : xbsIndex_r4/Reinterpret  force_arith_type=on  arith_type=Unsigned  force_bin_pt=on  bin_pt=8  block_type=cast
      settings: block_version=VER_STRING_GOES_HERE
  b0 : xbsIndex_r4/Slice  nbits=1  boolean_output=off  mode=Lower Bit Location + Width  bit1=0  base1=MSB of Input  bit0=0  base0=LSB of Input  dbl_ovrd=off  block_type=slice
      settings: block_version=VER_STRING_GOES_HERE
  b[0] : xbsIndex_r4/Slice  nbits=1  boolean_output=off  mode=Lower Bit Location + Width  bit1=0  base1=MSB of Input  bit0=0  base0=LSB of Input  dbl_ovrd=off  block_type=slice
      settings: block_version=VER_STRING_GOES_HERE
  "b[11:0]   " : xbsIndex_r4/Slice  nbits=12  boolean_output=off  mode=Lower Bit Location + Width  bit1=0  base1=MSB of Input  bit0=0  base0=LSB of Input  dbl_ovrd=off  block_type=slice
      settings: block_version=VER_STRING_GOES_HERE
  b[11:8] : xbsIndex_r4/Slice  nbits=4  boolean_output=off  mode=Lower Bit Location + Width  bit1=0  base1=MSB of Input  bit0=8  base0=LSB of Input  dbl_ovrd=off  block_type=slice
      settings: block_version=VER_STRING_GOES_HERE
  b[14:10] : xbsIndex_r4/Slice  nbits=5  boolean_output=off  mode=Lower Bit Location + Width  bit1=0  base1=MSB of Input  bit0=10  base0=LSB of Input  dbl_ovrd=off  block_type=slice
      settings: block_version=VER_STRING_GOES_HERE
  b[15:0] : xbsIndex_r4/Slice  nbits=16  boolean_output=off  mode=Lower Bit Location + Width  bit1=0  base1=MSB of Input  bit0=0  base0=LSB of Input  dbl_ovrd=off  block_type=slice
      settings: block_version=VER_STRING_GOES_HERE
  "b[15:0]    " : xbsIndex_r4/Slice  nbits=16  boolean_output=off  mode=Lower Bit Location + Width  bit1=0  base1=MSB of Input  bit0=0  base0=LSB of Input  dbl_ovrd=off  block_type=slice
      settings: block_version=VER_STRING_GOES_HERE
  b[15:0]x : xbsIndex_r4/Slice  nbits=16  boolean_output=off  mode=Lower Bit Location + Width  bit1=0  base1=MSB of Input  bit0=0  base0=LSB of Input  dbl_ovrd=off  block_type=slice
      settings: block_version=VER_STRING_GOES_HERE
  b[15:8] : xbsIndex_r4/Slice  nbits=8  boolean_output=off  mode=Lower Bit Location + Width  bit1=0  base1=MSB of Input  bit0=8  base0=LSB of Input  dbl_ovrd=off  block_type=slice
      settings: block_version=VER_STRING_GOES_HERE
  "b[16:12]   " : xbsIndex_r4/Slice  nbits=5  boolean_output=off  mode=Lower Bit Location + Width  bit1=0  base1=MSB of Input  bit0=12  base0=LSB of Input  dbl_ovrd=off  block_type=slice
      settings: block_version=VER_STRING_GOES_HERE
  b[17:8] 1 : xbsIndex_r4/Slice  nbits=10  boolean_output=off  mode=Lower Bit Location + Width  bit1=0  base1=MSB of Input  bit0=8  base0=LSB of Input  dbl_ovrd=off  block_type=slice
      settings: block_version=VER_STRING_GOES_HERE
  b[19:5] : xbsIndex_r4/Slice  nbits=15  boolean_output=off  mode=Lower Bit Location + Width  bit1=0  base1=MSB of Input  bit0=5  base0=LSB of Input  dbl_ovrd=off  block_type=slice
      settings: block_version=VER_STRING_GOES_HERE
  "b[21:8] " : xbsIndex_r4/Slice  nbits=14  boolean_output=off  mode=Lower Bit Location + Width  bit1=0  base1=MSB of Input  bit0=8  base0=LSB of Input  dbl_ovrd=off  block_type=slice
      settings: block_version=VER_STRING_GOES_HERE
  b[23:16] : xbsIndex_r4/Slice  nbits=8  boolean_output=off  mode=Lower Bit Location + Width  bit1=0  base1=MSB of Input  bit0=16  base0=LSB of Input  dbl_ovrd=off  block_type=slice
      settings: block_version=VER_STRING_GOES_HERE
  b[23:16]1 : xbsIndex_r4/Slice  nbits=8  boolean_output=off  mode=Lower Bit Location + Width  bit1=0  base1=MSB of Input  bit0=16  base0=LSB of Input  dbl_ovrd=off  block_type=slice
      settings: block_version=VER_STRING_GOES_HERE
  b[23:8] : xbsIndex_r4/Slice  nbits=16  boolean_output=off  mode=Lower Bit Location + Width  bit1=0  base1=MSB of Input  bit0=8  base0=LSB of Input  dbl_ovrd=off  block_type=slice
      settings: block_version=VER_STRING_GOES_HERE
  b[24:18] : xbsIndex_r4/Slice  nbits=7  boolean_output=off  mode=Lower Bit Location + Width  bit1=0  base1=MSB of Input  bit0=18  base0=LSB of Input  dbl_ovrd=off  block_type=slice
      settings: block_version=VER_STRING_GOES_HERE
  b[24:20] : xbsIndex_r4/Slice  nbits=5  boolean_output=off  mode=Lower Bit Location + Width  bit1=0  base1=MSB of Input  bit0=20  base0=LSB of Input  dbl_ovrd=off  block_type=slice
      settings: block_version=VER_STRING_GOES_HERE
  "b[25:22] " : xbsIndex_r4/Slice  nbits=4  boolean_output=off  mode=Lower Bit Location + Width  bit1=0  base1=MSB of Input  bit0=22  base0=LSB of Input  dbl_ovrd=off  block_type=slice
      settings: block_version=VER_STRING_GOES_HERE
  b[29:24] : xbsIndex_r4/Slice  nbits=6  boolean_output=off  mode=Lower Bit Location + Width  bit1=0  base1=MSB of Input  bit0=24  base0=LSB of Input  dbl_ovrd=off  block_type=slice
      settings: block_version=VER_STRING_GOES_HERE
  b[31:16] : xbsIndex_r4/Slice  nbits=16  boolean_output=off  mode=Lower Bit Location + Width  bit1=0  base1=MSB of Input  bit0=16  base0=LSB of Input  dbl_ovrd=off  block_type=slice
      settings: block_version=VER_STRING_GOES_HERE
  b[31:24] : xbsIndex_r4/Slice  nbits=8  boolean_output=off  mode=Lower Bit Location + Width  bit1=0  base1=MSB of Input  bit0=24  base0=LSB of Input  dbl_ovrd=off  block_type=slice
      settings: block_version=VER_STRING_GOES_HERE
  b[31:25] : xbsIndex_r4/Slice  nbits=7  boolean_output=off  mode=Lower Bit Location + Width  bit1=0  base1=MSB of Input  bit0=25  base0=LSB of Input  dbl_ovrd=off  block_type=slice
      settings: block_version=VER_STRING_GOES_HERE
  b[31:26] : xbsIndex_r4/Slice  nbits=6  boolean_output=off  mode=Lower Bit Location + Width  bit1=0  base1=MSB of Input  bit0=26  base0=LSB of Input  dbl_ovrd=off  block_type=slice
      settings: block_version=VER_STRING_GOES_HERE
  b[31] : xbsIndex_r4/Slice  nbits=1  boolean_output=off  mode=Lower Bit Location + Width  bit1=0  base1=MSB of Input  bit0=31  base0=LSB of Input  dbl_ovrd=off  block_type=slice
      settings: block_version=VER_STRING_GOES_HERE
  b[3:0] : xbsIndex_r4/Slice  nbits=4  boolean_output=off  mode=Lower Bit Location + Width  bit1=0  base1=MSB of Input  bit0=0  base0=LSB of Input  dbl_ovrd=off  block_type=slice
      settings: block_version=VER_STRING_GOES_HERE
  b[4:0] : xbsIndex_r4/Slice  nbits=5  boolean_output=off  mode=Lower Bit Location + Width  bit1=0  base1=MSB of Input  bit0=0  base0=LSB of Input  dbl_ovrd=off  block_type=slice
      settings: block_version=VER_STRING_GOES_HERE
  "b[4:0]  " : xbsIndex_r4/Slice  nbits=5  boolean_output=off  mode=Lower Bit Location + Width  bit1=0  base1=MSB of Input  bit0=0  base0=LSB of Input  dbl_ovrd=off  block_type=slice
      settings: block_version=VER_STRING_GOES_HERE
  b[7:0] : xbsIndex_r4/Slice  nbits=8  boolean_output=off  mode=Lower Bit Location + Width  bit1=0  base1=MSB of Input  bit0=0  base0=LSB of Input  dbl_ovrd=off  block_type=slice
      settings: block_version=VER_STRING_GOES_HERE
  "b[7:0] " : xbsIndex_r4/Slice  nbits=8  boolean_output=off  mode=Lower Bit Location + Width  bit1=0  base1=MSB of Input  bit0=0  base0=LSB of Input  dbl_ovrd=off  block_type=slice
      settings: block_version=VER_STRING_GOES_HERE
  b[7:0] 1 : xbsIndex_r4/Slice  nbits=8  boolean_output=off  mode=Lower Bit Location + Width  bit1=0  base1=MSB of Input  bit0=0  base0=LSB of Input  dbl_ovrd=off  block_type=slice
      settings: block_version=VER_STRING_GOES_HERE
  b[7:0] 2 : xbsIndex_r4/Slice  nbits=8  boolean_output=off  mode=Lower Bit Location + Width  bit1=0  base1=MSB of Input  bit0=0  base0=LSB of Input  dbl_ovrd=off  block_type=slice
      settings: block_version=VER_STRING_GOES_HERE
  b[7:0]1 : xbsIndex_r4/Slice  nbits=8  boolean_output=off  mode=Lower Bit Location + Width  bit1=0  base1=MSB of Input  bit0=0  base0=LSB of Input  dbl_ovrd=off  block_type=slice
      settings: block_version=VER_STRING_GOES_HERE
  b[9:5] : xbsIndex_r4/Slice  nbits=5  boolean_output=off  mode=Lower Bit Location + Width  bit1=0  base1=MSB of Input  bit0=5  base0=LSB of Input  dbl_ovrd=off  block_type=slice
      settings: block_version=VER_STRING_GOES_HERE
  dbg_gpio : xbsIndex_r4/Gateway Out  inherit_from_input=off  hdl_port=on  timing_constraint=None  locs_specified=off  LOCs={}  UseAsDAC=off  DACChannel='1'  block_type=gatewayout
  tag scope: CS_SW_TRIG=global, regRx_CCA_PostRx_Extension=global, regRx_CP_LEN=global, regRx_DSSS_PKT_BUF_SEL=global, regRx_DSSS_RX_CODE_CORR_THRESH=global, regRx_DSSS_RX_DESPREAD_DLY=global, regRx_DSSS_RX_TIMEOUT_BITS_TO_SFD=global, regRx_DSSS_RX_TIMEOUT_MIN_ONES=global, regRx_DSSS_RX_TIMEOUT_MIN_ONES_TOT=global, regRx_Debug_RSSI_Sel=global, regRx_Debug_RSSI_THRESH=global, regRx_FEC_SCALE_16QAM=global, regRx_FEC_SCALE_64QAM=global, regRx_FEC_SCALE_QPSK=global, regRx_FFT_OFFSET=global, regRx_FFT_SCALING=global, regRx_Global_Reset=global, regRx_LongCorr_RSSI_Thresh=global, regRx_LongCorr_Thresh_highSNR=global, regRx_LongCorr_Thresh_lowSNR=global, regRx_NUM_SC=global, regRx_OFDM_PKT_BUF_SEL=global, regRx_PKT_DET_RESET_EXT_DUR=global, regRx_PktBuf_Max_WriteAddr=global, regRx_PktBuf_Rx_Addr_Offset=global, regRx_PktBuf_Rx_H_Est_Offset=global, regRx_PktDet_Timout=global, regRx_RSSI_CS_THRESH=global
  wires:
  b[31:16]/1 -> Register11/1
  From Register1/1 -> b[7:0]/1, b[15:8]/1, b[23:16]/1, b[29:24]/1
  b[7:0]/1 -> Register4/1
  b[15:8]/1 -> Register5/1
  b[23:16]/1 -> Register6/1
  From Register2/1 -> b[0]/1
  b[0]/1 -> Register50/1
  From Register3/1 -> "b[4:0]  "/1, b[9:5]/1, b[14:10]/1
  b[29:24]/1 -> Register7/1
  [DSSS_FCS_GOOD] -> Concat/4
  [OFDM_FCS_GOOD] -> Concat/5
  Constant1/1 -> To Register/2
  To Register/1 -> Terminator/1
  From Register4/1 -> "b[7:0] "/1, "b[21:8] "/1, "b[25:22] "/1, b[31:26]/1
  "b[7:0] "/1 -> To_UFix8_7/1
  "b[21:8] "/1 -> To_UFix8_1/1
  To_UFix8_7/1 -> Down Sample16/1
  To_UFix8_1/1 -> Down Sample1/1
  Down Sample16/1 -> Register19/1
  Down Sample1/1 -> Register21/1
  b[15:0]/1 -> Register14/1
  "b[25:22] "/1 -> Down Sample2/1
  Down Sample2/1 -> Register23/1
  Sim Reset/1(Out1) -> ?
  From Register5/1 -> b[3:0]/1, b[11:8]/1, " b[23:16] "/1, " b[31:24]"/1
  b[3:0]/1 -> Register44/1
  From Register6/1 -> "b[11:0]   "/1, "b[16:12]   "/1, b[31:24]/1
  "b[11:0]   "/1 -> To_UFix8_2/1
  To_UFix8_2/1 -> Register37/1
  From Register8/1 -> "b[15:0]    "/1, b[31]/1
  "b[15:0]    "/1 -> Register33/1
  From Register9/1 -> b[7:0]1/1, b0/1
  b[7:0]1/1 -> Register1/1
  Register1/1 -> Register2/1
  Register2/1 -> Register3/1
  Register3/1 -> dbg_gpio/1
  b[4:0]/1 -> Register8/1
  Down Sample6/1 -> b[4:0]/1, b[19:5]/1, b[24:20]/1
  b[19:5]/1 -> Register9/1
  From Register/1 -> Down Sample6/1
  b[24:20]/1 -> Register10/1
  From Register7/1 -> Register48/1
  Register4/1 -> [regRx_NUM_SC]
  Register5/1 -> [regRx_CP_LEN]
  Register6/1 -> [regRx_FFT_OFFSET]
  Register7/1 -> [regRx_FFT_SCALING]
  Register8/1 -> Fanout1/1(In1)
  Register9/1 -> Fanout1/2(In2)
  Register10/1 -> Fanout1/3(In3)
  Register11/1 -> [regRx_LongCorr_Thresh_highSNR]
  b[31:26]/1 -> Register25/1
  From Register11/1 -> b[15:0]x/1, b[23:16]1/1
  b[15:0]x/1 -> [regRx_RSSI_CS_THRESH]
  b[23:16]1/1 -> [regRx_CCA_PostRx_Extension]
  b[11:8]/1 -> Register45/1
  Convert/1 -> To Register/1
  " b[23:16] "/1 -> Register46/1
  "b[4:0]  "/1 -> [regRx_FEC_SCALE_QPSK]
  b[9:5]/1 -> [regRx_FEC_SCALE_16QAM]
  b[14:10]/1 -> [regRx_FEC_SCALE_64QAM]
  b0/1 -> Register12/1
  Register12/1 -> [CS_SW_TRIG]
  Constant4/1 -> To Register2/2
  To Register2/1 -> Terminator2/1
  Convert2/1 -> To Register2/1
  Register13/1 -> [regRx_LongCorr_RSSI_Thresh]
  b[23:8]/1 -> Register13/1
  Register14/1 -> [regRx_LongCorr_Thresh_lowSNR]
  From Register14/1 -> b[15:0]/1, b[31:16]/1
  From Register12/1 -> b[7:0] 1/1, b[23:8]/1
  "2x "/1(2d) -> [regRx_PktDet_Timout]
  Register15/1 -> "2x "/1(d)
  b[7:0] 1/1 -> Register15/1
  " b[31:24]"/1 -> Register47/1
  [RSSI_POST_AGC_AB] -> Register56/1
  [ANTENNA_SEL] -> Concat/3
  Constant2/1 -> To Register3/2
  To Register3/1 -> Terminator3/1
  Convert3/1 -> To Register3/1
  [GAINS_POST_AGC] -> Register55/1
  Register16/1 -> Convert3/1
  Register17/1 -> Convert2/1
  b[31]/1 -> Register36/1
  Concat/1 -> Register51/1
  [PKTDET_STATUS_OFDM] -> Concat/2
  Constant5/1 -> To Register4/2
  To Register4/1 -> Terminator4/1
  Convert4/1 -> To Register4/1
  Register18/1 -> Convert4/1
  [RSSI_POST_AGC_CD] -> Register57/1
  Register19/1 -> Fanout/1(Corr_Thresh)
  Register21/1 -> Fanout/2(E_Thresh)
  Register23/1 -> Fanout/3(MinDur)
  Register33/1 -> Register34/1
  Register34/1 -> [regRx_Debug_RSSI_THRESH]
  Register35/1 -> [regRx_Debug_RSSI_Sel]
  Register36/1 -> Register35/1
  Register37/1 -> [regRx_DSSS_RX_CODE_CORR_THRESH]
  Register40/1 -> Register41/1
  Register41/1 -> [regRx_DSSS_RX_DESPREAD_DLY]
  Register44/1 -> [regRx_OFDM_PKT_BUF_SEL]
  Register45/1 -> [regRx_DSSS_PKT_BUF_SEL]
  Register46/1 -> [regRx_PktBuf_Rx_Addr_Offset]
  Register47/1 -> [regRx_PktBuf_Rx_H_Est_Offset]
  Register48/1 -> Register49/1
  Register49/1 -> Config Bits/1(32b)
  Register50/1 -> [regRx_Global_Reset]
  Register51/1 -> Register52/1
  Register52/1 -> Convert/1
  Register55/1 -> Register16/1
  Register56/1 -> Register17/1
  Register57/1 -> Register18/1
  Register25/1 -> Register26/1
  Register26/1 -> [regRx_PKT_DET_RESET_EXT_DUR]
  From Register13/1 -> b[7:0] 2/1, b[17:8] 1/1, b[24:18]/1, b[31:25]/1
  b[7:0] 2/1 -> To_UFix8_3/1
  To_UFix8_3/1 -> Down Sample3/1
  Down Sample3/1 -> Register20/1
  Register20/1 -> Fanout2/1(Corr_Thresh)
  b[17:8] 1/1 -> Down Sample4/1
  Down Sample4/1 -> Register22/1
  Register22/1 -> Fanout2/2(E_Thresh)
  [PKTDET_STATUS_DSSS] -> Concat/1
  "b[16:12]   "/1 -> Register40/1
  b[31:24]/1 -> Register24/1
  Register24/1 -> Register27/1
  Register27/1 -> [regRx_DSSS_RX_TIMEOUT_BITS_TO_SFD]
  b[24:18]/1 -> Down Sample5/1
  Down Sample5/1 -> Register28/1
  Register28/1 -> [regRx_DSSS_RX_TIMEOUT_MIN_ONES]
  b[31:25]/1 -> Down Sample7/1
  Down Sample7/1 -> Register29/1
  Register29/1 -> [regRx_DSSS_RX_TIMEOUT_MIN_ONES_TOT]
  From Register10/1 -> Register30/1
  Register30/1 -> Register31/1
  Register31/1 -> Register32/1
  Register32/1 -> [regRx_PktBuf_Max_WriteAddr]

== system wlan_phy_rx_pmd/Resets ==
  Convert1 : xbsIndex_r4/Convert  gui_display_data_type=Boolean  arith_type=Boolean  n_bits=16  bin_pt=14  float_type=Single  exp_bits=8  fraction_bits=24  quantization=Truncate  overflow=Wrap  en=off  latency=0  dbl_ovrd=off  pipeline=off  block_type=convert
  Pulse Generator : DiscretePulseGenerator  Period=1e9  PulseWidth=8  PhaseDelay=100
  tag scope: RX_GLOBAL_RESET=global
  wires:
  [regRx_Global_Reset] -> Convert1/1
  Convert1/1 -> [RX_GLOBAL_RESET]
